simulink model "TosAeb"  (R2021a)
settings: Solver=FixedStepDiscrete  StartTime=0.0  StopTime=inf  FixedStep=0.01  RelTol=1e-3
undefined symbols (not defined in the file or in its folder): ACCModSts, AccSts1, AEB_ObjectMovingOrientation, AEB_ObjectMovingState, CN_BTCActive, CN_CurrentGearR, CN_CurrentGearR_DHT, CN_PTCActive, CN_VDCActive, CN_VSC_EspOff, CN_VSC_FCW_AEB_Error, CN_VSC_FCW_AEB_NoDispay, CN_VSC_FCW_AEB_PerfmDgdtn, CN_VSC_FCW_AEB_TempUnAvlbl, CN_VSC_VehStandstill, CNaccelAvoidMax, CNaccelAvoidMin, CNbrakePedalPress, CNcomfortStrLeftAccelMax, CNcomfortStrLeftAccelMin, CNcomfortStrRightAccelMax, CNcomfortStrRightAccelMin, CNDrvDoorOpen, CNDrvModeEco, CNDrvModeNorm, CNDrvModeOther, CNDrvModeSport, CNDrvSBRreminder, CNNull, CNobjInvalidID, CNPassDoorOpen, CNPassSBRreminder, CNPEBSAccelNegInf, CNPEBSAccelNull, CNPEBSTimeInf, CNPEBSTimeNull, CNSTOPMAX, CNSTOPNULL, CNsubjWidth, CNSwtOFF, CNSwtON, CNTTCMAX, CNTTCNULL, CNVehConfigAEBCycNone, CNVehConfigAEBNone, CNVehConfigAEBPedNone, CNVehConfigFCWNone, CNVehConfigJANone, CollisionSeverity, FALSE, FCWSnvty, JACycObjCalcInfoDefaultParams, JAPedObjCalcInfoDefaultParams, JAVehObjCalcInfoDefaultParams, latCycObjCalcInfoDefaultParams, latPedObjCalcInfoDefaultParams, longObjCalcInfoDefaultParams, ModSts, movingStatus, ObjectType ... (+1112)
libraries: simulink x3031, AEBSFunctionLib x50, Ta_Library x33, AEBSStateMachineLib x23, Mylibrary x12, simulink_extras x7, ObjSelect_lib x3, dspstat3 x2
(part 58 of 102)

== system TosAeb/FcwAebJa_Step_sys/ALG_AEBSystem/ALG_AEBController/AEB_LongTgtInfoCalc/LongObjCalcInfoCalc/AEBS_LongCollisionAssesApp/collisionSeverityHighCalc/collisionSeverityHighCalc1/BrkSysAtribution ==
  FCWSnvty : Inport
  ObjectMovingState : Inport  Port=2
  subjVel : Inport  Port=3
  subjLongAccel : Inport  Port=4
  AccelGradientMO : Constant  Commented=on  Value=P_LongCA_SeverityHighAccelGredientMO
  AccelGradientSO : Constant  Commented=on  Value=P_LongCA_SeverityHighAccelGredientSO
  AccelMaxReudceMO : Constant  Commented=on  Value=P_LongCA_SeverityHighAccelMaxDecMO
  AccelMaxReudceSO : Constant  Commented=on  Value=P_LongCA_SeverityHighAccelMaxDecSO
  Bus Creator : BusCreator  Inputs=3
  Bus Selector : BusSelector  OutputSignals=ObjectMovingState,subjVel,subjLongAccel
  Bus Selector1 : BusSelector  OutputSignals=ObjectMovingState,subjVel,subjLongAccel
  Bus Selector2 : BusSelector  OutputSignals=ObjectMovingState,subjVel,subjLongAccel
  Bus Selector3 : BusSelector  OutputSignals=brkSysAtribution,tBrakeLatency
  FCWSnvtyEarly : Subsystem  TreatAsAtomicUnit=on
  FCWSnvtyLate : Subsystem  TreatAsAtomicUnit=on
  FCWSnvtyMid : Subsystem  TreatAsAtomicUnit=on
  Merge : Merge  Inputs=3
  Switch Case : SwitchCase  CaseConditions={FCWSnvty.HMISettingEarly,FCWSnvty.HMISettingLate}
  brkSysAtribution : Outport
  tBrakeLatency : Outport  Port=2
  wires:
  FCWSnvtyEarly/1(brkSys) -> Merge/1
  FCWSnvty/1 -> Switch Case/1
  Switch Case/1 -> FCWSnvtyEarly/Action
  subjLongAccel/1 -> Bus Creator/3  "subjLongAccel"
  ObjectMovingState/1 -> Bus Creator/2  "ObjectMovingState"
  subjVel/1 -> Bus Creator/1  "subjVel"
  Bus Creator/1 -> Bus Selector2/1, Bus Selector1/1, Bus Selector/1
  FCWSnvtyLate/1(brkSys) -> Merge/2
  FCWSnvtyMid/1(brkSys) -> Merge/3
  Switch Case/2 -> [FCWSnvtyLate]
  [FCWSnvtyLate] -> FCWSnvtyLate/Action
  Switch Case/3 -> [FCWSnvtyMid]
  [FCWSnvtyMid] -> FCWSnvtyMid/Action
  Merge/1 -> Bus Selector3/1
  Bus Selector3/1 -> brkSysAtribution/1  "<brkSysAtribution>"
  Bus Selector3/2 -> tBrakeLatency/1  "<tBrakeLatency>"
  Bus Selector/1 -> FCWSnvtyEarly/1(ObjectMovingState)  "<ObjectMovingState>"
  Bus Selector/2 -> FCWSnvtyEarly/2(subjVel)  "<subjVel>"
  Bus Selector/3 -> FCWSnvtyEarly/3(subjLongAccel)  "<subjLongAccel>"
  Bus Selector1/2 -> FCWSnvtyLate/2(subjVel)  "<subjVel>"
  Bus Selector1/1 -> FCWSnvtyLate/1(ObjectMovingState)  "<ObjectMovingState>"
  Bus Selector1/3 -> FCWSnvtyLate/3(subjLongAccel)  "<subjLongAccel>"
  Bus Selector2/1 -> FCWSnvtyMid/1(ObjectMovingState)  "<ObjectMovingState>"
  Bus Selector2/2 -> FCWSnvtyMid/2(subjVel)  "<subjVel>"
  Bus Selector2/3 -> FCWSnvtyMid/3(subjLongAccel)  "<subjLongAccel>"

== system TosAeb/FcwAebJa_Step_sys/ALG_AEBSystem/ALG_AEBController/AEB_LongTgtInfoCalc/LongObjCalcInfoCalc/AEBS_LongCollisionAssesApp/collisionSeverityHighCalc/collisionSeverityHighCalc1/CollisionAssessHighCalcVersion3 ==
  accelGradient : Inport
  accelMaxDec : Inport  Port=2
  velMaxReudce : Inport  Port=3
  tBrakeLatency : Inport  Port=4
  subjLongAccel : Inport  Port=5
  vxAbsObj : Inport  Port=6
  axAbsObj : Inport  Port=7
  subjVel : Inport  Port=8
  dxFrontBumper : Inport  Port=9
  dxSecure : Inport  Port=10
  CollisionAssessHighCalc1 : Subsystem  TreatAsAtomicUnit=on
  CollisionAssessHighCalc2 : Subsystem  Commented=on  TreatAsAtomicUnit=on
  CollisionAssessHighCalcInvalid : Subsystem
  If : If  IfExpression=u1
  If Action Subsystem : Subsystem  TreatAsAtomicUnit=on
  Merge : Merge
  collisionSeverityHighFlg : Outport
  wires:
  accelGradient/1 -> CollisionAssessHighCalc1/2(accelGradient), CollisionAssessHighCalcInvalid/1(accelGradient)  "aDtGradient"
  velMaxReudce/1 -> CollisionAssessHighCalc1/3(velMaxReudce)  "velMaxReudce"
  tBrakeLatency/1 -> CollisionAssessHighCalc1/4(tBrakeLatency)  "tBrakeLatency"
  axAbsObj/1 -> CollisionAssessHighCalc1/6(axAbsObj)  "axAbsObj"
  vxAbsObj/1 -> CollisionAssessHighCalc1/5(vxAbsObj)  "vxAbsObj"
  subjVel/1 -> CollisionAssessHighCalc1/7(subjVel)  "subjVel"
  subjLongAccel/1 -> CollisionAssessHighCalc1/8(subjLongAccel)  "subjLongAccel"
  dxSecure/1 -> CollisionAssessHighCalc1/10(dxSecure)  "dxSecure"
  dxFrontBumper/1 -> CollisionAssessHighCalc1/9(dxFrontBumper)  "dxFrontBumper"
  Merge/1 -> collisionSeverityHighFlg/1
  CollisionAssessHighCalc1/1(collisionSeverityHighFlg) -> Merge/2
  If Action Subsystem/1(collisionSeverityHighFlg) -> Merge/1
  CollisionAssessHighCalcInvalid/1(Invalid) -> If/1
  accelMaxDec/1 -> CollisionAssessHighCalc1/1(accelMaxDec), CollisionAssessHighCalcInvalid/2(accelMaxDec)
  If/2 -> CollisionAssessHighCalc1/Action
  If/1 -> If Action Subsystem/Action

== system TosAeb/FcwAebJa_Step_sys/ALG_AEBSystem/ALG_AEBController/AEB_LongTgtInfoCalc/LongObjCalcInfoCalc/AEBS_LongCollisionAssesApp/collisionSeverityHighCalc/collisionSeverityHighCalc1/dxSecureCalc ==
  vx : Inport
  DistSecure4collisionSeverityHigh : Lookup_n-D  NumberOfTableDimensions=1  BreakpointsForDimension1=P_AEB_LongCA_SeverityHighDistSecureX  BeginIndexSearchUsingPreviousIndexResult=on  Table=P_AEB_LongCA_SeverityHighDistSecureY  ExtrapMethod=Clip  InternalRulePriority=Speed  RndMeth=Simplest  InputSameDT=on
  dxSecure : Outport
  wires:
  DistSecure4collisionSeverityHigh/1 -> dxSecure/1  "dxSecure"
  vx/1 -> DistSecure4collisionSeverityHigh/1  "VelRelTargetObjCalc"

== system TosAeb/FcwAebJa_Step_sys/ALG_AEBSystem/ALG_AEBController/AEB_LongTgtInfoCalc/LongObjCalcInfoCalc/AEBS_LongCollisionAssesApp/collisionSeverityHighCalc/collisionSeverityHighCalc2/BrkSysAtribution ==
  ObjectMovingState : Inport
  AccelGradientMO : Constant  Value=PcollisionDgrLvl3AccelGredientMO
  AccelGradientSO : Constant  Value=PcollisionDgrLvl3AccelGredientSO
  AccelMaxReudceMO : Constant  Value=PcollisionDgrLvl3AccelMaxMO
  AccelMaxReudceSO : Constant  Value=PcollisionDgrLvl3AccelMaxSO
  Bus Creator : BusCreator  Inputs=3
  Bus Creator1 : BusCreator  Inputs=3
  Compare To Constant : simulink/Logic and Bit Operations/Compare To Constant  relop===  const=AEB_ObjectMovingState.Still  OutDataTypeStr=boolean
  Switch : Switch  Criteria=u2 ~= 0
  VelMaxReudceMO : Constant  Value=PcollisionDgrLvl3AccelMaxReduceMO
  VelMaxReudceSO : Constant  Value=PcollisionDgrLvl3AccelMaxReduceSO
  brkSysAtribution : Outport
  wires:
  VelMaxReudceSO/1 -> Bus Creator/1  "velMaxReudce"
  AccelMaxReudceSO/1 -> Bus Creator/2  "accelMaxReudce"
  AccelGradientSO/1 -> Bus Creator/3  "accelGradient"
  Bus Creator/1 -> Switch/1
  Switch/1 -> brkSysAtribution/1  "brkSysAtribution"
  Bus Creator1/1 -> Switch/3
  AccelGradientMO/1 -> Bus Creator1/3  "accelGradient"
  AccelMaxReudceMO/1 -> Bus Creator1/2  "accelMaxReudce"
  VelMaxReudceMO/1 -> Bus Creator1/1  "velMaxReudce"
  ObjectMovingState/1 -> Compare To Constant/1
  Compare To Constant/1 -> Switch/2

== system TosAeb/FcwAebJa_Step_sys/ALG_AEBSystem/ALG_AEBController/AEB_LongTgtInfoCalc/LongObjCalcInfoCalc/AEBS_LongCollisionAssesApp/collisionSeverityHighCalc/collisionSeverityHighCalc2/collisionSeverityHighFlgJudge ==
  collisionSeverityHighFlgPhase1 : Inport
  collisionSeverityHighFlgPhase2 : Inport  Port=2
  collisionSeverityHighFlgPhase3 : Inport  Port=3
  Logical Operator : Logic  Operator=OR  Inputs=3  AllPortsSameDT=off  OutDataTypeStr=boolean
  collisionSeverityHighFlg : Outport
  wires:
  collisionSeverityHighFlgPhase2/1 -> Logical Operator/2  "collisionSeverityHighFlgPhase2"
  collisionSeverityHighFlgPhase3/1 -> Logical Operator/3  "collisionSeverityHighFlgPhase3"
  Logical Operator/1 -> collisionSeverityHighFlg/1  "collisionSeverityHighFlg"
  collisionSeverityHighFlgPhase1/1 -> Logical Operator/1  "collisionSeverityHighFlgPhase1"

== system TosAeb/FcwAebJa_Step_sys/ALG_AEBSystem/ALG_AEBController/AEB_LongTgtInfoCalc/LongObjCalcInfoCalc/AEBS_LongCollisionAssesApp/collisionSeverityHighCalc/collisionSeverityHighCalc2/collisionSeverityHighPhase1 ==
  velMaxReudce : Inport
  dxFrontBumper : Inport  Port=2
  subjLongAccel : Inport  Port=3
  subjVel : Inport  Port=4
  ax : Inport  Port=5
  vxAbsObj : Inport  Port=6
  axAbsObj : Inport  Port=7
  vx : Inport  Port=8
  dxSecure : Inport  Port=9
  Bus Creator : BusCreator  Inputs=9
  Bus Creator1 : BusCreator  Inputs=4
  Bus Selector : BusSelector  OutputSignals=velMaxReudce,subjLongAccel,subjVel,vxAbsObj,axAbsObj
  Bus Selector1 : BusSelector  OutputSignals=subjVel,subjLongAccel,vxAbsObj,axAbsObj,dxFrontBumper,dxSecure
  CollisionSeverityHighPhase1Judge : Subsystem
  Phase1DistCalc : Subsystem
  Phase1TimeCalc : Subsystem
  collisionSeverityHighPhase1Out : Outport
  wires:
  Phase1TimeCalc/1(tWorkPhase1) -> Phase1DistCalc/1(tWorkPhase1), Bus Creator1/1  "tWorkPhase1"
  Phase1TimeCalc/2(tObjStopPhase1) -> Phase1DistCalc/2(tObjStopPhase1)
  Phase1DistCalc/1(distEgoPhase1) -> Bus Creator1/2, CollisionSeverityHighPhase1Judge/1(distEgophase1)  "distEgoPhase1"
  Phase1DistCalc/2(distTargetPhase1) -> [distTargetPhase1], CollisionSeverityHighPhase1Judge/2(distObjphase1)
  CollisionSeverityHighPhase1Judge/1(collisionSeverityHighFlgPhase1) -> Bus Creator1/3  "collisionSeverityHighFlgPhase1"
  Bus Creator/1 -> Bus Selector1/1, Bus Selector/1
  subjLongAccel/1 -> Bus Creator/3  "subjLongAccel"
  subjVel/1 -> Bus Creator/4  "subjVel"
  ax/1 -> Bus Creator/5  "ax"
  vxAbsObj/1 -> Bus Creator/6  "vxAbsObj"
  axAbsObj/1 -> Bus Creator/7  "axAbsObj"
  dxFrontBumper/1 -> Bus Creator/2  "dxFrontBumper"
  velMaxReudce/1 -> Bus Creator/1  "velMaxReudce"
  Bus Selector/1 -> Phase1TimeCalc/1(velMaxReudce)  "<velMaxReudce>"
  Bus Selector/2 -> Phase1TimeCalc/2(subjLongAccel)  "<subjLongAccel>"
  Bus Selector/3 -> Phase1TimeCalc/3(subjVel)  "<subjVel>"
  Bus Selector/4 -> Phase1TimeCalc/4(vxAbsObj)  "<vxAbsObj>"
  Bus Selector/5 -> Phase1TimeCalc/5(axAbsObj)  "<axAbsObj>"
  Bus Selector1/1 -> Phase1DistCalc/3(subjVel)  "<subjVel>"
  Bus Selector1/2 -> Phase1DistCalc/4(subjLongAccel)  "<subjLongAccel>"
  Bus Selector1/3 -> Phase1DistCalc/5(vxAbsObj)  "<vxAbsObj>"
  Bus Selector1/4 -> Phase1DistCalc/6(axAbsObj)  "<axAbsObj>"
  Bus Selector1/5 -> CollisionSeverityHighPhase1Judge/3(dxFrontBumper)  "<dxFrontBumper>"
  vx/1 -> Bus Creator/8  "vx"
  [distTargetPhase1] -> Bus Creator1/4  "distTargetPhase1"
  Bus Creator1/1 -> collisionSeverityHighPhase1Out/1  "collisionSeverityHighPhase1Out"
  dxSecure/1 -> Bus Creator/9  "dxSecure"
  Bus Selector1/6 -> CollisionSeverityHighPhase1Judge/4(dxSecure)  "<dxSecure>"

== system TosAeb/FcwAebJa_Step_sys/ALG_AEBSystem/ALG_AEBController/AEB_LongTgtInfoCalc/LongObjCalcInfoCalc/AEBS_LongCollisionAssesApp/collisionSeverityHighCalc/collisionSeverityHighCalc2/collisionSeverityHighPhase2 ==
  tWorkphase1 : Inport
  distTargetphase1 : Inport  Port=2
  distEgophase1 : Inport  Port=3
  subjVel : Inport  Port=4
  subjLongAccel : Inport  Port=5
  vxAbsObj : Inport  Port=6
  axAbsObj : Inport  Port=7
  dxFrontBumper : Inport  Port=8
  velMaxReduce : Inport  Port=9
  accelGradient : Inport  Port=10
  dxSecure : Inport  Port=11
  accelMaxReudce : Inport  Port=12
  Bus Creator : BusCreator  Inputs=5
  Bus Creator1 : BusCreator  Inputs=5
  Bus Selector : BusSelector  OutputSignals=subjAccelWorkPhase1,subjVelWorkPhase1,vxAbsWorkPhase1Target,axAbsWorkPhase1Target
  Bus Selector1 : BusSelector  OutputSignals=subjVelWorkPhase1,subjAccelWorkPhase1,vxAbsWorkPhase1Target,axAbsWorkPhase1Target
  DistPhase2Calc : Subsystem
  EgoObjAfterPhase1 : Subsystem
  Phase2TimeCalc : Subsystem
  collisionSeverityHighPhase2FlgCalc : Subsystem
  collisionSeverityHighPhase2Out : Outport
  wires:
  subjVel/1 -> EgoObjAfterPhase1/4(subjVel)  "subjVel"
  tWorkphase1/1 -> EgoObjAfterPhase1/1(tWorkphase1)  "tWorkphase1"
  subjLongAccel/1 -> EgoObjAfterPhase1/5(subjLongAccel)  "subjAccel"
  vxAbsObj/1 -> EgoObjAfterPhase1/6(vxAbsObj)  "vxAbsObj"
  axAbsObj/1 -> EgoObjAfterPhase1/7(axAbsObj)  "axAbsObj"
  distTargetphase1/1 -> EgoObjAfterPhase1/2(distTargetphase1)  "distTargetphase1"
  distEgophase1/1 -> EgoObjAfterPhase1/3(distEgophase1)  "distEgophase1"
  dxFrontBumper/1 -> EgoObjAfterPhase1/8(dxFrontBumper)  "dxFrontBumper"
  DistPhase2Calc/1(distEgophase2) -> collisionSeverityHighPhase2FlgCalc/1(distEgophase2), Bus Creator1/2  "distEgophase2"
  DistPhase2Calc/2(distTargetphase2) -> collisionSeverityHighPhase2FlgCalc/2(distTargetPhase2), [distTargetphase2]
  collisionSeverityHighPhase2FlgCalc/1(collisionSeverityHighPhase2) -> Bus Creator1/3  "collisionSeverityHighFlgPhase2"
  Phase2TimeCalc/1(tWorkPhase2) -> [tWorkPhase2], DistPhase2Calc/6(tWorkPhase2)
  Phase2TimeCalc/2(tObjStopWorkPhase2) -> DistPhase2Calc/7(tObjStopWorkPhase2)
  EgoObjAfterPhase1/2(subjLongAccelWorkPhase1) -> Bus Creator/2  "subjAccelWorkPhase1"
  EgoObjAfterPhase1/1(subjVelWorkPhase1) -> Bus Creator/1  "subjVelWorkPhase1"
  EgoObjAfterPhase1/3(vxAbsWorkPhase1Target) -> Bus Creator/3  "vxAbsWorkPhase1Target"
  EgoObjAfterPhase1/4(axAbsWorkPhase1Target) -> Bus Creator/4  "axAbsWorkPhase1Target"
  EgoObjAfterPhase1/5(distRelWorkPhase1) -> [distRelWorkPhase1], Bus Creator/5  "distRelWorkPhase1"
  Bus Creator/1 -> Bus Creator1/1, Bus Selector1/1, Bus Selector/1  "EgoObjDisVelAccelAfterPhase1"
  Bus Selector/4 -> Phase2TimeCalc/4(axAbsWorkPhase1Target)  "<axAbsWorkPhase1Target>"
  Bus Selector/3 -> Phase2TimeCalc/3(vxAbsWorkPhase1Target)  "<vxAbsWorkPhase1Target>"
  Bus Selector1/1 -> DistPhase2Calc/2(subVelWorkPhase1)  "<subjVelWorkPhase1>"
  Bus Selector1/2 -> DistPhase2Calc/3(subjLongAccelWorkPhase1)  "<subjAccelWorkPhase1>"
  Bus Selector1/3 -> DistPhase2Calc/4(vxAbsWorkPhase1Target)  "<vxAbsWorkPhase1Target>"
  Bus Selector1/4 -> DistPhase2Calc/5(axAbsWorkPhase1Target)  "<axAbsWorkPhase1Target>"
  velMaxReduce/1 -> Phase2TimeCalc/5(velMaxReudce)
  accelGradient/1 -> Phase2TimeCalc/6(accelGradient), [accelGradient]
  dxSecure/1 -> collisionSeverityHighPhase2FlgCalc/4(dxSecure)  "dxSecure"
  [distRelWorkPhase1] -> collisionSeverityHighPhase2FlgCalc/3(distRelWorkPhase1)  "distRelWorkPhase1"
  [tWorkPhase2] -> Bus Creator1/4  "tWorkPhase2"
  [distTargetphase2] -> Bus Creator1/5  "distTargetphase2"
  Bus Creator1/1 -> collisionSeverityHighPhase2Out/1  "collisionSeverityHighPhase2Out"
  accelMaxReudce/1 -> Phase2TimeCalc/7(accelMaxReudce)
  [accelGradient] -> DistPhase2Calc/1(accelGradient)
  Bus Selector/1 -> Phase2TimeCalc/1(subjLongAccelWorkPhase1)  "<subjAccelWorkPhase1>"
  Bus Selector/2 -> Phase2TimeCalc/2(subjVelWorkPhase1)  "<subjVelWorkPhase1>"

== system TosAeb/FcwAebJa_Step_sys/ALG_AEBSystem/ALG_AEBController/AEB_LongTgtInfoCalc/LongObjCalcInfoCalc/AEBS_LongCollisionAssesApp/collisionSeverityHighCalc/collisionSeverityHighCalc2/collisionSeverityHighPhase3 ==
  subjVelWorkPhase1 : Inport
  "tWorkPhase2 " : Inport  Port=2
  axAbsWorkPhase1Target : Inport  Port=3
  subjLongAccelWorkPhase1 : Inport  Port=4
  vxAbsWorkPhase1Target : Inport  Port=5
  distTargetPhase2 : Inport  Port=6
  dxRelWorkPhase1 : Inport  Port=7
  distEgoPhase2 : Inport  Port=8
  accelGradient : Inport  Port=9
  accelMaxReduce : Inport  Port=10
  dxSecure : Inport  Port=11
  velMaxReudce : Inport  Port=12
  Bus Creator : BusCreator  Inputs=5
  Bus Selector : BusSelector  OutputSignals=subjVeltWorkPhase2,subjAccelWorkPhase2,vxAbsWorkPhase2Target,axAbsWorkPhase2Target
  Bus Selector1 : BusSelector  OutputSignals=subjVeltWorkPhase2,vxAbsWorkPhase2Target,axAbsWorkPhase2Target
  CollisionSeverityHighPhase3Calc : Subsystem
  DistPhase3Calc : Subsystem
  EgoObjAfterPhase2 : Subsystem
  Phase3TimeCalc : Subsystem
  collisionSeverityHighPhase3 : Outport
  wires:
  CollisionSeverityHighPhase3Calc/1(collisionSeverityHighPhase3) -> collisionSeverityHighPhase3/1  "collisionDangerLevel3Phase3"
  subjVelWorkPhase1/1 -> EgoObjAfterPhase2/1(subjVelWorkPhase1)
  "tWorkPhase2 "/1 -> EgoObjAfterPhase2/2(tWorkPhase2)
  subjLongAccelWorkPhase1/1 -> EgoObjAfterPhase2/4(subjLongAccelWorkPhase1)
  axAbsWorkPhase1Target/1 -> EgoObjAfterPhase2/3(axAbsWorkPhase1Target)
  vxAbsWorkPhase1Target/1 -> EgoObjAfterPhase2/5(vxAbsWorkPhase1Target)
  accelGradient/1 -> EgoObjAfterPhase2/9(accelGradient)
  distTargetPhase2/1 -> EgoObjAfterPhase2/6(distTargetPhase2)
  distEgoPhase2/1 -> EgoObjAfterPhase2/8(distEgoPhase2)
  dxRelWorkPhase1/1 -> EgoObjAfterPhase2/7(dxRelWorkPhase1)  "dxRelWorkPhase1"
  Phase3TimeCalc/2(tObjStopWorkPhase3) -> DistPhase3Calc/6(tObjStopWorkPhase3)
  Phase3TimeCalc/1(tWorkPhase3) -> DistPhase3Calc/5(tWorkPhase3)
  DistPhase3Calc/1(distEgophase3) -> CollisionSeverityHighPhase3Calc/1(distEgoPhase3)  "distEgophase3"
  DistPhase3Calc/2(distTargetPhase3) -> CollisionSeverityHighPhase3Calc/2(distTargetPhase3)
  EgoObjAfterPhase2/1(subjVelWorkPhase2) -> Bus Creator/1  "subjVeltWorkPhase2"
  EgoObjAfterPhase2/2(subjLongAccelWorkPhase2) -> Bus Creator/2  "subjAccelWorkPhase2"
  EgoObjAfterPhase2/3(vxAbsWorkPhase2Target) -> Bus Creator/3  "vxAbsWorkPhase2Target"
  EgoObjAfterPhase2/4(axAbsWorkPhase2Target) -> Bus Creator/4  "axAbsWorkPhase2Target"
  EgoObjAfterPhase2/5(distRelWorkPhase2) -> Bus Creator/5, [distRelWorkPhase2]  "distRelWorkPhase2"
  Bus Creator/1 -> Bus Selector1/1, Bus Selector/1
  Bus Selector/3 -> Phase3TimeCalc/3(vxAbsWorkPhase2Target)  "<vxAbsWorkPhase2Target>"
  Bus Selector/2 -> Phase3TimeCalc/2(subjLongAccelWorkPhase2)  "<subjAccelWorkPhase2>"
  Bus Selector/4 -> Phase3TimeCalc/4(axAbsWorkPhase2Target)  "<axAbsWorkPhase2Target>"
  Bus Selector/1 -> Phase3TimeCalc/1(subjVelWorkPhase2)  "<subjVeltWorkPhase2>"
  Bus Selector1/2 -> DistPhase3Calc/3(vxAbsWorkPhase2Target)  "<vxAbsWorkPhase2Target>"
  [accelMaxReduce] -> DistPhase3Calc/1(subjLongAccelWorkPhase2)
  Bus Selector1/3 -> DistPhase3Calc/4(axAbsWorkPhase2Target)  "<axAbsWorkPhase2Target>"
  Bus Selector1/1 -> DistPhase3Calc/2(subVelWorkPhase2)  "<subjVeltWorkPhase2>"
  [distRelWorkPhase2] -> CollisionSeverityHighPhase3Calc/3(distRelWorkPhase2)  "distRelWorkPhase2"
  dxSecure/1 -> CollisionSeverityHighPhase3Calc/4(dxSecure)  "dxSecure"
  velMaxReudce/1 -> Phase3TimeCalc/6(velMaxReudce)  "velMaxReudce"
  accelMaxReduce/1 -> [accelMaxReduce], Phase3TimeCalc/5(accelMaxReduce)  "accelMaxReduce"

== system TosAeb/FcwAebJa_Step_sys/ALG_AEBSystem/ALG_AEBController/AEB_LongTgtInfoCalc/LongObjCalcInfoCalc/AEBS_LongCollisionAssesApp/collisionSeverityHighCalc/collisionSeverityHighCalc2/dxSecureCalc ==
  vx : Inport
  DistSecure4collisionSeverityHigh : Lookup_n-D  NumberOfTableDimensions=1  BreakpointsForDimension1=PcollisionDgrLvl3DistSecureX  BeginIndexSearchUsingPreviousIndexResult=on  Table=PcollisionDgrLvl3DistSecureY  ExtrapMethod=Clip  InternalRulePriority=Speed  RndMeth=Simplest  InputSameDT=on
  dxSecure : Outport
  wires:
  vx/1 -> DistSecure4collisionSeverityHigh/1  "VelRelTargetObjCalc"
  DistSecure4collisionSeverityHigh/1 -> dxSecure/1  "dxSecure"

== system TosAeb/AEB_Objsel/TA_Processor/AEB_ObjSelect/TargetInfoCheck/Data_precess/TA_EffectCalculate/Obj_FilterFlgCalculate/JA_ObjFilter/CollisionProbCalc/If Action Subsystem ==
  Action Port : ActionPort  ActionPortLabel=if(u1)
  Constant : Constant  Value=0  OutDataTypeStr=boolean
  ObjColliFlg : Outport
  wires:
  Constant/1 -> ObjColliFlg/1

== system TosAeb/AEB_Objsel/TA_Processor/AEB_ObjSelect/TargetInfoCheck/Data_precess/TA_EffectCalculate/Obj_FilterFlgCalculate/JA_ObjFilter/CollisionProbCalc/ObjIsInvalidCalc ==
  ObjectID : Inport
  Compare To Constant : simulink/Logic and Bit Operations/Compare To Constant  relop===  const=0  OutDataTypeStr=boolean
  ObjIsInvalid : Outport
  wires:
  Compare To Constant/1 -> ObjIsInvalid/1
  ObjectID/1 -> Compare To Constant/1

== system TosAeb/AEB_Objsel/TA_Processor/AEB_ObjSelect/TargetInfoCheck/Data_precess/TA_EffectCalculate/Obj_FilterFlgCalculate/JA_ObjFilter/CollisionProbCalc/Subsystem ==
  tPredTime : Inport  OutDataTypeStr=single
  subjVel : Inport  Port=2  OutDataTypeStr=single
  subjLongAccel : Inport  Port=3  OutDataTypeStr=single
  subjLatAccel : Inport  Port=4  OutDataTypeStr=single
  subjYawRate : Inport  Port=5  OutDataTypeStr=single
  ObjectYawAngle : Inport  Port=6  OutDataTypeStr=single
  ObjectLength : Inport  Port=7  OutDataTypeStr=single
  ObjectWidth : Inport  Port=8  OutDataTypeStr=single
  dx : Inport  Port=9  OutDataTypeStr=single
  dy : Inport  Port=10  OutDataTypeStr=single
  ay : Inport  Port=11  OutDataTypeStr=single
  vy : Inport  Port=12  OutDataTypeStr=single
  vxAbsObj : Inport  Port=13  OutDataTypeStr=single
  axAbsObj : Inport  Port=14  OutDataTypeStr=single
  PahEstimn : Inport  Port=15
  Action Port : ActionPort  ActionPortLabel=else
  ObjBoundingBox : Subsystem
  ObjBoundingBoxRebuild : Subsystem
  ObjPreReferPointCalc : Subsystem
  PreCrossFlgCalcFlow : Stateflow chart  TreatAsAtomicUnit=on
  PreRefPointEgoCalc : Subsystem  Commented=on
  SubjBoundingBox : Subsystem
  Subsystem : Subsystem
  ay_Filt : Subsystem
  sincosCoefCalc : Subsystem
  sincosCoefCalc1 : Subsystem
  ObjColliFlg : Outport
  wires:
  [subjLatAccel] -> ObjPreReferPointCalc/6(subjLatAccel)
  subjYawRate/1 -> PreRefPointEgoCalc/5(subjYawRate)
  subjLatAccel/1 -> [subjLatAccel], PreRefPointEgoCalc/4(subjLatAccel)
  subjVel/1 -> PreRefPointEgoCalc/1(subjVel)
  subjLongAccel/1 -> PreRefPointEgoCalc/3(subjLongAccel)
  axAbsObj/1 -> ObjPreReferPointCalc/4(axAbsObj)
  vxAbsObj/1 -> ObjPreReferPointCalc/2(vxAbsObj)
  dx/1 -> ObjPreReferPointCalc/1(dx)
  ObjectYawAngle/1 -> ObjPreReferPointCalc/9(ObjectYawAngle)
  PreCrossFlgCalcFlow/1(preCrossFlg) -> ObjColliFlg/1
  ObjBoundingBox/2(objDyPre) -> ObjBoundingBoxRebuild/4(objDyPre)
  ObjBoundingBox/1(objDxPre) -> ObjBoundingBoxRebuild/3(objDxPre)
  SubjBoundingBox/2(subjDyPre) -> ObjBoundingBoxRebuild/2(subjDyPre)
  SubjBoundingBox/1(subjDxPre) -> ObjBoundingBoxRebuild/1(subjDxPre)
  sincosCoefCalc1/1(sincosCoef) -> ObjBoundingBox/5(sincosCoef)
  ObjectLength/1 -> ObjBoundingBox/1(ObjLengthCorr)
  ObjectWidth/1 -> ObjBoundingBox/2(ObjWidthCorr)
  sincosCoefCalc/1(sincosCoef) -> SubjBoundingBox/3(sincosCoef)
  dy/1 -> ObjPreReferPointCalc/8(dy)
  vy/1 -> ObjPreReferPointCalc/5(vy)
  tPredTime/1 -> ObjPreReferPointCalc/3(tPredTime), PreRefPointEgoCalc/2(tPredTime), Subsystem/1(tPredTime)
  ay/1 -> ay_Filt/1(ay)
  ay_Filt/1(ay_Filt) -> ObjPreReferPointCalc/7(ay)
  PahEstimn/1 -> Subsystem/2(PahEstimn)
  Subsystem/1(PredHostPosnLgt) -> SubjBoundingBox/1(dxPreRefPoint)
  Subsystem/2(PredHostPosnLat) -> SubjBoundingBox/2(dyPreRefPoint)
  Subsystem/3(PredHostHeading) -> sincosCoefCalc/1(angle)
  ObjPreReferPointCalc/1(dxPreRefPointObj) -> ObjBoundingBox/3(dxPreRefPointObj)
  ObjPreReferPointCalc/2(dyPreRefPointObj) -> ObjBoundingBox/4(dyPreRefPointObj)
  ObjPreReferPointCalc/3(YawAnglePreObj) -> sincosCoefCalc1/1(angle)
  ObjBoundingBoxRebuild/1(Out1) -> PreCrossFlgCalcFlow/1(subjDxPre)
  ObjBoundingBoxRebuild/2(Out2) -> PreCrossFlgCalcFlow/2(subjDyPre)
  ObjBoundingBoxRebuild/3(Out3) -> PreCrossFlgCalcFlow/3(objDxPre)
  ObjBoundingBoxRebuild/4(Out4) -> PreCrossFlgCalcFlow/4(objDyPre)

== system TosAeb/AEB_Objsel/TA_Processor/AEB_ObjSelect/TargetInfoCheck/Data_precess/TA_EffectCalculate/Obj_FilterFlgCalculate/JA_ObjFilter/JAObjEnable/JAObjEnableJudge ==
  TA_ObsType : Inport
  TA_ObsVxAbs : Inport  Port=2
  TA_ObsConfidence : Inport  Port=3
  JAObjAbsVxEnable : Subsystem
  JAObjConfEnable : Subsystem
  JAObjTypeEnable : Subsystem
  Logical Operator : Logic  Inputs=3  AllPortsSameDT=off  OutDataTypeStr=boolean
  JAObjEnable : Outport
  wires:
  TA_ObsVxAbs/1 -> JAObjAbsVxEnable/2(TA_ObsVxAbs)
  JAObjAbsVxEnable/1(JAObjAbsVxEnable) -> Logical Operator/2
  Logical Operator/1 -> JAObjEnable/1
  TA_ObsConfidence/1 -> JAObjConfEnable/1(TA_ObsConfidence)
  TA_ObsType/1 -> JAObjAbsVxEnable/1(TA_ObsType), JAObjTypeEnable/1(TA_ObsType)
  JAObjConfEnable/1(JAObjConfEnable) -> Logical Operator/3
  JAObjTypeEnable/1(JAObjTypeEnable) -> Logical Operator/1

== system TosAeb/AEB_Objsel/TA_Processor/AEB_ObjSelect/TargetInfoCheck/Data_precess/TA_EffectCalculate/Obj_FilterFlgCalculate/Lat_ObjFilter/Lat_Objelse/Lat_ObjPre ==
  Obj_Type : Inport
  Obj_Oreintation : Inport  Port=2
  Obj_State : Inport  Port=3
  Obj_Vx : Inport  Port=4
  Obj_Vy : Inport  Port=5
  dx : Inport  Port=6
  dy : Inport  Port=7
  ax : Inport  Port=8
  ay : Inport  Port=9
  Width : Inport  Port=10
  ObsVyAbs : Inport  Port=11
  Subj_Vx : Inport  Port=12
  YawRate : Inport  Port=13
  LatObj_StateJudgment : Subsystem
  LatObj_TypeJudgment : Subsystem
  Subj_State : Subsystem
  Subsystem : Subsystem
  Type_State : Subsystem
  LatObj_Valid : Outport
  Lat_ObjIntersect : Outport  Port=2
  Lat_TTR : Outport  Port=3
  wires:
  Obj_Type/1 -> LatObj_TypeJudgment/1(Obj_Type)
  Obj_Oreintation/1 -> [Oreintation], LatObj_StateJudgment/1(Obj_Oreintation)
  Obj_State/1 -> LatObj_StateJudgment/2(Obj_State)
  Obj_Vx/1 -> [vx], LatObj_StateJudgment/3(Obj_Vx)
  Obj_Vy/1 -> [vy]
  Subj_Vx/1 -> Subj_State/1(Subj_Vx), LatObj_StateJudgment/4(Subj_Vx)
  LatObj_TypeJudgment/1(Type_Enable) -> Type_State/1(Type_Enable)
  LatObj_StateJudgment/1(State_Enable) -> Type_State/2(State_Enable)
  Type_State/1(LatObj_Valid) -> LatObj_Valid/1
  Subj_State/1(Subj_State) -> Type_State/3(Subj_State)
  dx/1 -> Subsystem/1(dx)
  dy/1 -> Subsystem/2(dy)
  ax/1 -> Subsystem/3(ax)
  ay/1 -> Subsystem/4(ay)
  [vx] -> Subsystem/7(vx)
  [vy] -> Subsystem/8(vy)
  Width/1 -> Subsystem/5(Width)
  [Oreintation] -> Subsystem/6(Oreintation)
  Subsystem/1(Lat_ObjIntersect) -> Lat_ObjIntersect/1
  Subsystem/2(Lat_TTR) -> Lat_TTR/1
  ObsVyAbs/1 -> LatObj_StateJudgment/5(ObsVyAbs)
  YawRate/1 -> Subj_State/2(YawRate)

== system TosAeb/AEB_Objsel/TA_Processor/AEB_ObjSelect/TargetInfoCheck/Data_precess/TA_EffectCalculate/Obj_FilterFlgCalculate/Long_ObjFilter/Info_Calculate/ObjWeight_Invalid ==
  Action Port : ActionPort  ActionPortLabel=if(u1 > 0)
  Constant : Constant  Value=255  OutDataTypeStr=uint8
  Long_ObjWeightCoef : Outport
  wires:
  Constant/1 -> Long_ObjWeightCoef/1

== system TosAeb/AEB_Objsel/TA_Processor/AEB_ObjSelect/TargetInfoCheck/Data_precess/TA_EffectCalculate/Obj_FilterFlgCalculate/Long_ObjFilter/Info_Calculate/ObjWeight_Jugement ==
  Obj_WeightCoef : Inport
  Obj_Oreintation : Inport  Port=2
  Obj_Accy : Inport  Port=3
  Obj_Accx : Inport  Port=4
  Obj_VyStd : Inport  Port=5
  Obj_Vy : Inport  Port=6
  Obj_VxStd : Inport  Port=7
  Obj_Vx : Inport  Port=8
  Obj_State : Inport  Port=9
  Obj_HeadAg : Inport  Port=10
  Subj_Vx : Inport  Port=11
  Subj_Yaw : Inport  Port=12
  Subj_Ay : Inport  Port=13
  Subj_Decel : Inport  Port=14
  AEB_State : Inport  Port=15
  Action Port : ActionPort  ActionPortLabel=else
  Compare To Constant : simulink/Logic and Bit Operations/Compare To Constant  relop===  const=3  OutDataTypeStr=boolean
  Compare To Constant1 : simulink/Logic and Bit Operations/Compare To Constant  relop===  const=4  OutDataTypeStr=boolean
  Compare To Constant3 : simulink/Logic and Bit Operations/Compare To Constant  relop=>=  const=1  OutDataTypeStr=boolean
  Compare To Constant4 : simulink/Logic and Bit Operations/Compare To Constant  relop=>=  const=1  OutDataTypeStr=boolean
  Constant : Constant  Value=255  OutDataTypeStr=uint8
  Constant1 : Constant  Value=0  OutDataTypeStr=boolean
  Constant2 : Constant  Value=0  OutDataTypeStr=boolean
  Logical Operator : Logic  Operator=OR  AllPortsSameDT=off  OutDataTypeStr=boolean
  Logical Operator1 : Logic  Operator=OR  Inputs=4  AllPortsSameDT=off  OutDataTypeStr=boolean
  Subsystem : Subsystem
  Switch : Switch  Criteria=u2 > Threshold
  Terminator{,1..5} (6 alike) : Terminator
  Long_ObjWeightCoef : Outport
  wires:
  Obj_Oreintation/1 -> Compare To Constant1/1, Compare To Constant/1
  Compare To Constant/1 -> Logical Operator/1
  Compare To Constant1/1 -> Logical Operator/2
  Subj_Ay/1 -> Terminator1/1
  Subj_Decel/1 -> Terminator2/1
  Obj_Accy/1 -> Terminator3/1
  Obj_Accx/1 -> Terminator4/1
  Obj_VxStd/1 -> Compare To Constant4/1
  Obj_VyStd/1 -> Compare To Constant3/1
  Obj_Vy/1 -> Subsystem/1(Obj_Vy)
  Obj_Vx/1 -> Subsystem/2(Obj_Vx)
  Subj_Vx/1 -> Subsystem/3(Subj_Vx)
  Subj_Yaw/1 -> Subsystem/4(Subj_Yaw)
  Logical Operator/1 -> Logical Operator1/1
  Compare To Constant3/1 -> Terminator/1
  Compare To Constant4/1 -> Terminator5/1
  Subsystem/1(Spd_Valid) -> Logical Operator1/4
  Logical Operator1/1 -> Switch/2
  Constant/1 -> Switch/1
  Obj_WeightCoef/1 -> Switch/3
  Switch/1 -> Long_ObjWeightCoef/1
  Obj_State/1 -> Subsystem/5(State)
  AEB_State/1 -> Subsystem/6(AEB_State)
  Obj_HeadAg/1 -> Subsystem/7(Obj_HeadAg)
  Constant1/1 -> Logical Operator1/2
  Constant2/1 -> Logical Operator1/3

== system TosAeb/AEB_Objsel/TA_Processor/AEB_ObjSelect/TargetInfoCheck/Data_precess/TA_EffectCalculate/Obj_FilterFlgCalculate/Long_ObjFilter/Info_Calculate/Subsystem ==
  Obj_WeightCoef : Inport
  Compare To Constant : simulink/Logic and Bit Operations/Compare To Constant  relop===  const=255  OutDataTypeStr=boolean
  Data Type Conversion : DataTypeConversion
  Obj_Coeflg : Outport
  wires:
  Obj_WeightCoef/1 -> Compare To Constant/1
  Compare To Constant/1 -> Data Type Conversion/1
  Data Type Conversion/1 -> Obj_Coeflg/1

== system TosAeb/AEB_Objsel/TA_Processor/AEB_ObjSelect/TargetInfoCheck/Data_precess/TA_EffectCalculate/Obj_FilterFlgCalculate/Long_ObjFilter/OverlapCalculate/BoudingBoxCalculate_Else ==
  TimeToReachLgt : Inport
  PahEstimn : Inport  Port=2
  TA_ObjInfo : Inport  Port=3
  VehSelfData : Inport  Port=4
  Action Port : ActionPort  ActionPortLabel=else
  Overlap_FlgCalculate : Subsystem
  PosnatTTI : Subsystem
  Boudingbox_Calculate : Outport
  wires:
  PosnatTTI/2(PosnLatHost) -> Overlap_FlgCalculate/2(PosnLatHost)
  PosnatTTI/1(PosnLgtHost) -> Overlap_FlgCalculate/1(PosnLgtHost)
  PosnatTTI/6(Obj_PreHeading) -> Overlap_FlgCalculate/6(Obj_PreHeading)
  PosnatTTI/9(Obj_Length) -> Overlap_FlgCalculate/9(Obj_Length)
  VehSelfData/1 -> PosnatTTI/4(VehSelfData)
  PosnatTTI/10(Obj_Width) -> Overlap_FlgCalculate/10(Obj_Width)
  PosnatTTI/3(HeadingHost) -> Overlap_FlgCalculate/3(HeadingHost)
  TA_ObjInfo/1 -> PosnatTTI/3(TA_ObjInfo)
  PosnatTTI/4(Obj_LongPosn) -> Overlap_FlgCalculate/4(Obj_LongPosn)
  TimeToReachLgt/1 -> PosnatTTI/1(TimeToReachLgt)
  PosnatTTI/5(Obj_LatPosn) -> Overlap_FlgCalculate/5(Obj_LatPosn)
  PahEstimn/1 -> PosnatTTI/2(PahEstimn)
  PosnatTTI/8(Relative_LatPosn) -> Overlap_FlgCalculate/8(Relative_LatPosn)
  PosnatTTI/7(Relative_LongPosn) -> Overlap_FlgCalculate/7(Relative_LongPosn)
  Overlap_FlgCalculate/1(Boudingbox_Calculate) -> Boudingbox_Calculate/1
  PosnatTTI/11(AEB_State) -> Overlap_FlgCalculate/11(AEB_State)

== system TosAeb/AEB_Objsel/TA_Processor/AEB_ObjSelect/TargetInfoCheck/Data_precess/TA_EffectCalculate/Obj_FilterFlgCalculate/Long_ObjFilter/OverlapCalculate/BoudingBoxCalculate_if ==
  Action Port : ActionPort  ActionPortLabel=if(u1 > 0)
  Constant : Constant  Value=0  OutDataTypeStr=boolean
  Boudingbox_Calculate : Outport
  wires:
  Constant/1 -> Boudingbox_Calculate/1

== system TosAeb/AEB_Objsel/TA_Processor/AEB_ObjSelect/TargetInfoCheck/Data_precess/TA_EffectCalculate/Obj_FilterFlgCalculate/Long_ObjFilter/OverlapCalculate/Subsystem ==
  Obj_WeightCoef : Inport
  Compare To Constant : simulink/Logic and Bit Operations/Compare To Constant  relop===  const=255  OutDataTypeStr=boolean
  Data Type Conversion : DataTypeConversion
  Obj_Coeflg : Outport
  wires:
  Obj_WeightCoef/1 -> Compare To Constant/1
  Compare To Constant/1 -> Data Type Conversion/1
  Data Type Conversion/1 -> Obj_Coeflg/1

== system TosAeb/AEB_Objsel/TA_Processor/AEB_ObjSelect/TargetInfoCheck/Data_precess/TA_EffectCalculate/Obj_FilterFlgCalculate/Long_ObjFilter/TTR_Calculate/CalculateTTRAndTTM_else ==
  Long_ObjWeightCoef : Inport
  TA_ObjInfo : Inport  Port=2
  VehSelfData : Inport  Port=3
  Action Port : ActionPort  ActionPortLabel=else
  Bus Selector1 : BusSelector  OutputSignals=VRel,ARel
  LongitudinalTTRComputer : Subsystem
  ModifyTTRAtSameSpeedAndAcceleration : Subsystem
  Obj_AbsInfo : Subsystem
  SetCorrectSign : Subsystem
  Subsystem : Subsystem
  Long_ObjWeightCoefTTI : Outport
  TimeToReachLgt : Outport  Port=2
  wires:
  [FarFront] -> SetCorrectSign/4(FarEdgeDistance)
  [CloseFront] -> SetCorrectSign/3(CloseEdgeDistance)
  LongitudinalTTRComputer/2(SolutionExists) -> SetCorrectSign/2(SolutionExists)
  Bus Selector1/2 -> ModifyTTRAtSameSpeedAndAcceleration/4(ARel)  "<ARel>"
  Bus Selector1/1 -> ModifyTTRAtSameSpeedAndAcceleration/3(VRel)  "<VRel>"
  [MotionFront] -> Bus Selector1/1
  [FarFront] -> ModifyTTRAtSameSpeedAndAcceleration/2(FarEdgeDistance)
  [CloseFront] -> ModifyTTRAtSameSpeedAndAcceleration/1(CloseEdgeDistance)
  Obj_AbsInfo/2(MotionDataRearClosest) -> LongitudinalTTRComputer/2(MotionDataRearClosest)
  Obj_AbsInfo/1(MotionDataFrontClosest) -> [MotionFront], LongitudinalTTRComputer/1(MotionDataFrontClosest)
  Obj_AbsInfo/5(CloseEdgeDistanceRearClosest) -> LongitudinalTTRComputer/5(EdgeDistanceRearClosest)
  Obj_AbsInfo/4(CloseEdgeDistanceFrontClosest) -> [CloseFront], LongitudinalTTRComputer/4(EdgeDistanceFrontClosest)
  [CloseFront] -> LongitudinalTTRComputer/6(PosnLgt)
  Obj_AbsInfo/6(FarEdgeDistanceFrontClosest) -> [FarFront]
  ModifyTTRAtSameSpeedAndAcceleration/2(TimeToReach) -> TimeToReachLgt/1  "TimeToReachLgt"
  LongitudinalTTRComputer/1(TimeToIntersection) -> SetCorrectSign/1(TTI_in)
  SetCorrectSign/1(TTI_out) -> ModifyTTRAtSameSpeedAndAcceleration/5(TimeToReachIn)
  VehSelfData/1 -> [VehSelfData], Obj_AbsInfo/2(VehSelfData)
  TA_ObjInfo/1 -> [TA_ObjInfo], Obj_AbsInfo/1(TA_ObjInfo)
  Obj_AbsInfo/3(StoppingData) -> LongitudinalTTRComputer/3(StoppingData)
  Long_ObjWeightCoef/1 -> Subsystem/1(Long_ObjWeightCoef)
  Subsystem/1(Long_ObjWeightCoefTTI) -> Long_ObjWeightCoefTTI/1  "Long_ObjWeightCoefTTI"
  ModifyTTRAtSameSpeedAndAcceleration/1(Valid_Time) -> Subsystem/4(TimeToReach)
  [VehSelfData] -> LongitudinalTTRComputer/7(VehSelfData)
  [TA_ObjInfo] -> Subsystem/2(TA_ObjInfo)
  [VehSelfData] -> Subsystem/3(VehSelfData)

== system TosAeb/AEB_Objsel/TA_Processor/AEB_ObjSelect/TargetInfoCheck/Data_precess/TA_EffectCalculate/Obj_FilterFlgCalculate/Long_ObjFilter/TTR_Calculate/CalculateTTRAndTTM_if ==
  Action Port : ActionPort  ActionPortLabel=if(u1 > 0)
  Constant : Constant  Value=255  OutDataTypeStr=uint8
  Constant1 : Constant  Value=P_TA_TTIUpperLimiPositive
  Long_ObjWeightCoefTTI : Outport
  TimeToReachLgt : Outport  Port=2
  wires:
  Constant/1 -> Long_ObjWeightCoefTTI/1
  Constant1/1 -> TimeToReachLgt/1  "TimeToReachLgt"

== system TosAeb/AEB_Objsel/TA_Processor/AEB_ObjSelect/TargetInfoCheck/Data_precess/TA_EffectCalculate/Obj_FilterFlgCalculate/Long_ObjFilter/TTR_Calculate/Subsystem ==
  Obj_WeightCoef : Inport
  Compare To Constant : simulink/Logic and Bit Operations/Compare To Constant  relop===  const=255  OutDataTypeStr=boolean
  Data Type Conversion : DataTypeConversion
  Obj_Coeflg : Outport
  wires:
  Obj_WeightCoef/1 -> Compare To Constant/1
  Compare To Constant/1 -> Data Type Conversion/1
  Data Type Conversion/1 -> Obj_Coeflg/1

== system TosAeb/AEB_Objsel/TA_Processor/AEB_ObjSelect/TargetInfoCheck/Data_precess/TA_EffectCalculate/ValideateObj/ObjPos_Calculate/ObjPos_Calculateelse/InPath_Cal ==
  TA_ObjLength : Inport
  TA_ObjWidth : Inport  Port=2
  TA_ObjDx : Inport  Port=3
  TA_ObjDy : Inport  Port=4
  TA_Objvy : Inport  Port=5
  TA_HeadingAnlge : Inport  Port=6
  Obj_AbsAccly : Inport  Port=7
  Obj_AbsSpdy : Inport  Port=8
  TA_ObjVx : Inport  Port=9
  PathEstimn : Inport  Port=10
  Overlap_Ratedelay : Inport  Port=11
  Constant : Constant  Value=P_TA_PrmForVehWidth
  Constant1 : Constant  Value=P_TA_PrmForObjDisThrd
  Constant2 : Constant  Value=P_TA_PrmForVehWidth
  Data Type Conversion9 : DataTypeConversion
  DetermineInPath : Subsystem
  Position_InPath : MATLAB Function  TreatAsAtomicUnit=on
  Overlap_Current : Outport
  Overlap_Prediction : Outport  Port=2
  Overlap_Rate : Outport  Port=3
  wires:
  TA_ObjLength/1 -> Position_InPath/1(Obj_Lenth)
  TA_ObjWidth/1 -> Position_InPath/2(Obj_Width), [Obj_Width]
  TA_ObjDx/1 -> Position_InPath/3(Obj_Dx), [Obj_dx]
  TA_HeadingAnlge/1 -> Position_InPath/6(Obj_Heading)
  Constant/1 -> Position_InPath/7(Subj_Width)
  Constant1/1 -> Position_InPath/8(P_TA_PrmForObjDisThrd)
  Position_InPath/1(Overlap_Flg) -> Overlap_Current/1
  PathEstimn/1 -> DetermineInPath/3(PathEstimn)
  Constant2/1 -> DetermineInPath/8(HostWidth)
  [Obj_Width] -> DetermineInPath/7(ObjWidth)
  [Obj_dx] -> DetermineInPath/6(PosnLgt)
  Obj_AbsAccly/1 -> DetermineInPath/1(ALat)
  Obj_AbsSpdy/1 -> DetermineInPath/2(VLat)
  DetermineInPath/1(InPath) -> Overlap_Prediction/1
  Data Type Conversion9/1 -> Position_InPath/9(Overlap_delay)
  TA_ObjDy/1 -> [Obj_dy], Position_InPath/4(Obj_Dy)
  TA_Objvy/1 -> Position_InPath/5(Obj_vy)
  [Obj_dy] -> DetermineInPath/5(PosnLat)
  TA_ObjVx/1 -> DetermineInPath/4(VLgtRel)
  Position_InPath/2(Overlap_Rate) -> Overlap_Rate/1
  Overlap_Ratedelay/1 -> Data Type Conversion9/1

== system TosAeb/AEB_Objsel/TA_Processor/AEB_ObjSelect/TargetInfoCheck/Data_precess/TA_EffectCalculate/ValideateObj/ValidObj_PreProcess/If Action Subsystem1/CheckBehavior ==
  note: % 0：unknown 1:Preceeding 2:Crossing_to_Left
      | % 3: Crossing_to_Right 4:Cut_in 5:Cut_Out
      | % 6: Oncoming 7: LTAP 8:RTAP
      | 滤除对向来车
  note: 0x0: Unkown
      | 0x1: Oncoming
      | 0x2: Preceding
      | 0x3: Cut_in
      | 0x4: Cut_out
      | 0x5: CROSSING
      | 0x6: LTAP
      | 0x7: RTAP
      | 领目版本
  TA_ObjBehavior : Inport
  TA_Objvx : Inport  Port=2
  Veh_LongSpd_mps : Inport  Port=3
  TA_ObsVxAbs : Inport  Port=4
  Constant : Constant  Value=0  OutDataTypeStr=uint8
  Constant1 : Constant  Value=P_TA_ObjBehaviorOnComingPara  OutDataTypeStr=single
  Constant2 : Constant  Value=6  OutDataTypeStr=uint8
  Constant3 : Constant  Value=0  OutDataTypeStr=uint8
  Logical Operator : Logic  Operator=OR  AllPortsSameDT=off  OutDataTypeStr=boolean
  Logical Operator1 : Logic  Operator=OR  AllPortsSameDT=off  OutDataTypeStr=boolean
  Logical Operator2 : Logic  Operator=NOT  AllPortsSameDT=off  OutDataTypeStr=boolean
  Logical Operator3 : Logic  AllPortsSameDT=off  OutDataTypeStr=boolean
  Relational Operator : RelationalOperator  Operator===  OutDataTypeStr=boolean  RndMeth=Simplest
  Relational Operator1 : RelationalOperator  Operator=<=  OutDataTypeStr=boolean  RndMeth=Simplest
  Relational Operator2 : RelationalOperator  Operator===  OutDataTypeStr=boolean  RndMeth=Simplest
  Relational Operator3 : RelationalOperator  OutDataTypeStr=boolean  RndMeth=Simplest
  Terminator : Terminator
  Obj_BehaviorEnable : Outport
  wires:
  TA_ObjBehavior/1 -> Relational Operator2/1, Relational Operator/1
  Constant/1 -> Relational Operator/2
  TA_Objvx/1 -> Relational Operator3/2
  Constant1/1 -> Relational Operator1/2
  Constant2/1 -> Relational Operator2/2
  Relational Operator1/1 -> Logical Operator3/2
  Relational Operator/1 -> Logical Operator1/1
  Logical Operator/1 -> Logical Operator2/1
  Constant3/1 -> Relational Operator3/1
  Relational Operator3/1 -> Logical Operator3/1
  Logical Operator3/1 -> Logical Operator/2
  TA_ObsVxAbs/1 -> Relational Operator1/1
  Relational Operator2/1 -> Logical Operator/1
  Veh_LongSpd_mps/1 -> Terminator/1
  Logical Operator1/1 -> Obj_BehaviorEnable/1
  Logical Operator2/1 -> Logical Operator1/2

== system TosAeb/AEB_Objsel/TA_Processor/AEB_ObjSelect/TargetInfoCheck/Data_precess/TA_EffectCalculate/ValideateObj/ValidObj_PreProcess/If Action Subsystem1/CheckPosition ==
  note: 20
  note: 120
  TA_Obsdx : Inport
  TA_Obsdy : Inport  Port=2
  Constant : Constant  Value=P_TA_ObjdxRangetheMin
  Constant1 : Constant  Value=P_TA_ObjdxRangetheMax
  Constant2 : Constant  Value=P_TA_ObjdyRangetheMin
  Constant3 : Constant  Value=P_TA_ObjdyRangetheMax
  Logical Operator : Logic  Inputs=4  AllPortsSameDT=off  OutDataTypeStr=boolean
  Relational Operator : RelationalOperator  Operator=<=  OutDataTypeStr=boolean  RndMeth=Simplest
  Relational Operator1 : RelationalOperator  OutDataTypeStr=boolean  RndMeth=Simplest
  Relational Operator2 : RelationalOperator  Operator=<=  OutDataTypeStr=boolean  RndMeth=Simplest
  Relational Operator3 : RelationalOperator  OutDataTypeStr=boolean  RndMeth=Simplest
  Subsystem : Subsystem
  Obj_PosEnable : Outport
  wires:
  Constant1/1 -> Relational Operator/2
  TA_Obsdx/1 -> Subsystem/1(ObsDx)
  Constant/1 -> Relational Operator1/2
  Constant2/1 -> Relational Operator3/2
  TA_Obsdy/1 -> Relational Operator2/1, Relational Operator3/1
  Constant3/1 -> Relational Operator2/2
  Relational Operator/1 -> Logical Operator/1
  Relational Operator1/1 -> Logical Operator/2
  Relational Operator2/1 -> Logical Operator/3
  Relational Operator3/1 -> Logical Operator/4
  Logical Operator/1 -> Obj_PosEnable/1
  Subsystem/1(Obsdx) -> Relational Operator1/1, Relational Operator/1

== system TosAeb/AEB_Objsel/TA_Processor/AEB_ObjSelect/TargetInfoCheck/Data_precess/TA_EffectCalculate/ValideateObj/ValidObj_PreProcess/If Action Subsystem1/CheckQuality ==
  note: 0:小于50%
      | 1:50%~70%
      | 2:70%~85%
      | 3:85%~100%
  TA_ObsQuality : Inport
  Compare To Constant : simulink/Logic and Bit Operations/Compare To Constant  relop=>=  const=1  OutDataTypeStr=boolean
  Obj_QualityEnable : Outport
  wires:
  TA_ObsQuality/1 -> Compare To Constant/1
  Compare To Constant/1 -> Obj_QualityEnable/1

== system TosAeb/AEB_Objsel/TA_Processor/AEB_ObjSelect/TargetInfoCheck/Data_precess/TA_EffectCalculate/ValideateObj/ValidObj_PreProcess/If Action Subsystem1/CheckType ==
  note: 0x0: Unclear
      | 0x1: Bus
      | 0x2: Car
      | 0x3: Truck
      | 0x4: Motor Bike
      | 0x5: Bicycle
      | 0x6: Pedestrian
      | 0x7: Animals
  TA_ObsType : Inport
  Bicycle : Subsystem
  Bus : Subsystem
  Car : Subsystem
  Invalid : Subsystem
  Logical Operator : Logic  Operator=OR  Inputs=6  AllPortsSameDT=off  OutDataTypeStr=boolean
  Logical Operator1 : Logic  AllPortsSameDT=off  OutDataTypeStr=boolean
  MotorBike : Subsystem
  Pedestrain : Subsystem
  Truck : Subsystem
  Obj_TpyeEnable : Outport
  wires:
  TA_ObsType/1 -> MotorBike/1(TA_ObsType), Bicycle/1(TA_ObsType), Pedestrain/1(TA_ObjType), Invalid/1(TA_ObjType), Bus/1(TA_ObsType), Car/1(TA_ObsType), Truck/1(TA_ObsType)
  Truck/1(Truck_Flg) -> Logical Operator/1
  Car/1(Car_Flg) -> Logical Operator/2
  Bus/1(Bus_Flg) -> Logical Operator/3
  MotorBike/1(MotorBike) -> Logical Operator/4
  Bicycle/1(Bicycle_Flg) -> Logical Operator/5
  Pedestrain/1(Pedestrian_Flg) -> Logical Operator/6
  Logical Operator/1 -> Logical Operator1/1
  Invalid/1(Invalid_Flg) -> Logical Operator1/2
  Logical Operator1/1 -> Obj_TpyeEnable/1

== system TosAeb/AEB_Objsel/TA_Processor/PreProcessor/ObjectPrecessor/TAObjInfoCalc/For Each Subsystem/Info_Check1/BasicInfo_check/AbsSpd/AbsVx_correct ==
  ObsVelXAbs_s : Inport
  Vhe_Spd : Inport  Port=2
  Obj_VxCr : Inport  Port=3
  Abs : Abs
  Add : Sum
  Add1 : Sum  Inputs=+-
  Compare To Constant : simulink/Logic and Bit Operations/Compare To Constant  relop=<=  const=2  OutDataTypeStr=boolean
  Switch : Switch  Criteria=u2 > Threshold
  Fus_ObjVxAbs : Outport
  wires:
  Vhe_Spd/1 -> Add/1
  Obj_VxCr/1 -> Add/2
  Add/1 -> Switch/3, Add1/2
  ObsVelXAbs_s/1 -> Fus_ObjVxAbs/1, Switch/1, Add1/1
  Add1/1 -> Abs/1
  Compare To Constant/1 -> Switch/2
  Abs/1 -> Compare To Constant/1

== system TosAeb/AEB_Objsel/TA_Processor/PreProcessor/ObjectPrecessor/TAObjInfoCalc/For Each Subsystem/Info_Check1/BasicInfo_check/AbsSpd/AbsVy_correct ==
  ObsVelYAbs_s : Inport
  Veh_YawRate : Inport  Port=2
  Obj_VyCr : Inport  Port=3
  Abs : Abs
  Add : Sum
  Add1 : Sum  Inputs=+-
  Compare To Constant : simulink/Logic and Bit Operations/Compare To Constant  relop=<=  const=1  OutDataTypeStr=boolean
  Constant : Constant  Value=P_TA_PrmForVehRearAxleDisttoFrnt
  Product : Product
  Switch : Switch  Criteria=u2 > Threshold
  Fus_ObjVyAbs : Outport
  wires:
  Constant/1 -> Product/2
  Veh_YawRate/1 -> Product/1
  Product/1 -> Add/1
  Obj_VyCr/1 -> Add/2
  Add/1 -> Switch/3, Add1/2
  ObsVelYAbs_s/1 -> Fus_ObjVyAbs/1, Switch/1, Add1/1
  Add1/1 -> Abs/1
  Abs/1 -> Compare To Constant/1
  Compare To Constant/1 -> Switch/2

== system TosAeb/AEB_Objsel/TA_Processor/PreProcessor/ObjectPrecessor/TAObjInfoCalc/For Each Subsystem/Info_Check1/BasicInfo_check/AccCorrect/Acc_XY ==
  ObsAccY_s : Inport
  Obj_vy : Inport  Port=2
  ObsAccX_s : Inport  Port=3
  Obj_Vx : Inport  Port=4
  Veh_AccX : Inport  Port=5
  Veh_AccY : Inport  Port=6
  Veh_AEBSta : Inport  Port=7
  Add : Sum  Inputs=+-
  Add1 : Sum  Inputs=+-
  Compare To Constant : simulink/Logic and Bit Operations/Compare To Constant  relop===  const=0  OutDataTypeStr=boolean
  Constant : Constant  Value=0  OutDataTypeStr=single
  Constant1 : Constant  Value=0  OutDataTypeStr=single
  Constant2 : Constant  Value=P_TA_ObjAccCalculateCalebration
  Constant3 : Constant  Value=P_TA_ObjAccCalculateCalebration
  Constant4 : Constant  Value=0.2  OutDataTypeStr=single
  LowPassFilter1 : Ta_Library/LowPassFilter  SystemSampleTime=-1  Latency=0  AutoFrameSizeCalculation=off
  Subsystem : Subsystem
  Subsystem1 : Subsystem
  Switch : Switch  Criteria=u2 > Threshold
  Switch1 : Switch  Criteria=u2 > Threshold
  Switch2 : Switch  Criteria=u2 ~= 0
  Switch3 : Switch  Criteria=u2 ~= 0
  Fus_ObjAy : Outport
  Fus_ObjAx : Outport  Port=2
  wires:
  Switch1/1 -> Switch2/1
  Switch/1 -> Switch3/1
  Constant3/1 -> Switch1/2
  Constant2/1 -> Switch/2
  Add1/1 -> Subsystem1/1(ObsAccX_s), Switch1/1
  Add/1 -> Subsystem/1(ObsAccY_s), Switch/1
  Obj_vy/1 -> Subsystem/2(Obj_vy)
  Subsystem/1(ObsAy_Correct) -> Switch/3
  Obj_Vx/1 -> Subsystem1/2(Obj_vX)
  Subsystem1/1(ObsAX_Correct) -> Switch1/3
  Veh_AEBSta/1 -> Compare To Constant/1
  Compare To Constant/1 -> [Aeb_state]
  ObsAccX_s/1 -> Add1/1
  Veh_AccX/1 -> Add1/2
  [Aeb_state] -> Switch2/2
  Constant/1 -> Switch2/3
  Switch2/1 -> Fus_ObjAx/1
  [Aeb_state] -> Switch3/2
  Constant1/1 -> Switch3/3
  ObsAccY_s/1 -> Add/1
  Switch3/1 -> Fus_ObjAy/1
  Veh_AccY/1 -> LowPassFilter1/2
  LowPassFilter1/1 -> Add/2
  Constant4/1 -> LowPassFilter1/1

== system TosAeb/AEB_Objsel/TA_Processor/PreProcessor/ObjectPrecessor/TAObjInfoCalc/For Each Subsystem/Info_Check1/BasicInfo_check/DxCorrect/Subsystem ==
  Obj_ID : Inport
  Compare To Constant : simulink/Logic and Bit Operations/Compare To Constant  relop=<=  const=0  OutDataTypeStr=boolean
  Constant : Constant  Value=P_TA_ObjAddAttrCalValue
  Constant1 : Constant  OutDataTypeStr=boolean
  Data Type Conversion : DataTypeConversion
  Logical Operator : Logic  Operator=OR  AllPortsSameDT=off  OutDataTypeStr=boolean
  Relational Operator : RelationalOperator  Operator=~=  OutDataTypeStr=boolean  RndMeth=Simplest
  Switch : Switch  Criteria=u2 > Threshold
  Unit Delay : UnitDelay  SampleTime=-1  HasFrameUpgradeWarning=on
  Obj_Invalid : Outport
  wires:
  Switch/1 -> Data Type Conversion/1
  Constant1/1 -> Switch/3
  Constant/1 -> Switch/2
  Logical Operator/1 -> Switch/1
  Relational Operator/1 -> Logical Operator/2
  Compare To Constant/1 -> Logical Operator/1
  Data Type Conversion/1 -> Obj_Invalid/1
  Unit Delay/1 -> Relational Operator/2
  Obj_ID/1 -> Unit Delay/1, Compare To Constant/1, Relational Operator/1

== system TosAeb/AEB_Objsel/TA_Processor/PreProcessor/ObjectPrecessor/TAObjInfoCalc/For Each Subsystem/Info_Check1/BasicInfo_check/DxCorrect/dx_charge ==
  Fus_ObjDx_Single : Inport
  Fus_ObjDxStd : Inport  Port=2
  Fus_ObjVx_Single : Inport  Port=3
  Veh_AEBFunState : Inport  Port=4
  Action Port : ActionPort
  Dx_Flag : Subsystem
  Signal Conversion : SignalConversion  OverrideOpt=off
  Signal Conversion2 : SignalConversion  OverrideOpt=off
  dx_change : Subsystem
  Fus_ObjDx : Outport
  Dx_CorrectFlg : Outport  Port=2
  wires:
  Signal Conversion2/1 -> Dx_CorrectFlg/1
  Signal Conversion/1 -> Fus_ObjDx/1
  Veh_AEBFunState/1 -> Dx_Flag/2(Veh_AEBFunState)
  Fus_ObjDx_Single/1 -> Dx_Flag/4(Fus_ObjDx_Single), dx_change/2(Fus_ObjDx_Single)
  Fus_ObjVx_Single/1 -> Dx_Flag/1(Fus_ObjVx_Single)
  dx_change/1(Fus_ObjDx) -> Signal Conversion/1
  Dx_Flag/1(Dx_CorrectFlg) -> dx_change/1(Dx_CorrectFlg), Signal Conversion2/1
  Fus_ObjDxStd/1 -> Dx_Flag/3(Fus_ObjDxStd)

== system TosAeb/AEB_Objsel/TA_Processor/PreProcessor/ObjectPrecessor/TAObjInfoCalc/For Each Subsystem/Info_Check1/BasicInfo_check/DxCorrect/dx_nocharge ==
  Fus_ObjDx_Single : Inport
  Action Port : ActionPort
  Constant3 : Constant  Value=0  OutDataTypeStr=boolean
  Signal Conversion : SignalConversion  OverrideOpt=off
  Fus_ObjDx : Outport
  Dx_CorrectFlg : Outport  Port=2
  wires:
  Signal Conversion/1 -> Fus_ObjDx/1
  Constant3/1 -> Dx_CorrectFlg/1
  Fus_ObjDx_Single/1 -> Signal Conversion/1
